simulink model "basic_test"  (R2013b) (continued)
== system basic_test == (continued)
      mask help:
      | Fold adders into DSPs: Causes adders to be absorbed into DSP blocks (supported in Virtex5)
      | Adder implementation: Cores using Fabric or DSP48 or behavioral HDL
      mask init: same as basic_test/pfb_fir_real0
      settings: AttributesFormatString=taps=4, add_latency=1
      mask Help: eval('xlWeb(''http://casper.berkeley.edu/wiki/Pfb_fir_real'')')
      mask Callback TotalTaps:
      | %num_taps = eval(get_param(gcb, 'TotalTaps'));
      | %old_vec = eval(get_param(gcb, 'mult_spec'));=-0l
      | 
      | %vec = 2.*ones(1,num_taps);
      | %old_size = length(old_vec);
      | 
      | %if(old_size <= num_taps),
      | %    new_size = old_size;
      | %else,
      | %    new_size = num_taps;
      | %end
      | 
      | %for n = 1:new_size, vec(n) = old_vec(n); end
      | %set_param(gcb, 'mult_spec', mat2str(vec));
      mask TypeOptions WindowType: bartlett | barthannwin | blackman | blackmanharris | bohamwin | chebwin | flattopwin | gausswin | hamming | hann | kaiser | nuttallwin | parzenwin | rectwin | tukeywin | triang | userwindow
      mask TypeOptions quantization: Truncate | Round  (unbiased: +/- Inf) | Round  (unbiased: Even Values)
      mask TypeOptions adder_imp: Behavioral | Fabric | DSP48
  pipeline : Subsystem  AncestorBlock=casper_library_delays/pipeline  mask(pipeline){latency=1}
      mask help:
      | An explicitly laid-out delay line to avoid mapping to SRL16s
      | for use in pipelining to help achieve timing closure.
      mask init:
      | pipeline_init(gcb,'latency',latency);
      settings: AttributesFormatString=Latency = %<latency>
      mask Display:
      | port_label('input', 1, 'd');
      | port_label('output', 1, 'q');
      | disp(['Z^-', num2str(latency)]);
  pipeline{1..7,9..12,14..21,25..29,93,256..263,275..276} (35 alike) : casper_library_delays/pipeline  latency=3
      settings: AttributesFormatString=Latency = %<latency>
  pipeline127 : Subsystem  AncestorBlock=casper_library_delays/pipeline  mask(pipeline){latency=2}
      mask help:
      | An explicitly laid-out delay line to avoid mapping to SRL16s
      | for use in pipelining to help achieve timing closure.
      mask init:
      | pipeline_init(gcb,'latency',latency);
      settings: AttributesFormatString=Latency = %<latency>
      mask Display:
      | port_label('input', 1, 'd');
      | port_label('output', 1, 'q');
      | disp(['Z^-', num2str(latency)]);
  pipeline128 : Subsystem  AncestorBlock=casper_library_delays/pipeline  mask(pipeline){latency=2}
      mask help:
      | An explicitly laid-out delay line to avoid mapping to SRL16s
      | for use in pipelining to help achieve timing closure.
      mask init:
      | pipeline_init(gcb,'latency',latency);
      settings: AttributesFormatString=Latency = %<latency>
      mask Display:
      | port_label('input', 1, 'd');
      | port_label('output', 1, 'q');
      | disp(['Z^-', num2str(latency)]);
  pipeline13 : Subsystem  AncestorBlock=casper_library_delays/pipeline  mask(pipeline){latency=1}
      mask help:
      | An explicitly laid-out delay line to avoid mapping to SRL16s
      | for use in pipelining to help achieve timing closure.
      mask init:
      | pipeline_init(gcb,'latency',latency);
      settings: AttributesFormatString=Latency = %<latency>
      mask Display:
      | port_label('input', 1, 'd');
      | port_label('output', 1, 'q');
      | disp(['Z^-', num2str(latency)]);
  pipeline22 : Subsystem  AncestorBlock=casper_library_delays/pipeline  mask(pipeline){latency=2}
      mask help:
      | An explicitly laid-out delay line to avoid mapping to SRL16s
      | for use in pipelining to help achieve timing closure.
      mask init:
      | pipeline_init(gcb,'latency',latency);
      settings: AttributesFormatString=Latency = %<latency>
      mask Display:
      | port_label('input', 1, 'd');
      | port_label('output', 1, 'q');
      | disp(['Z^-', num2str(latency)]);
  pipeline23 : Subsystem  AncestorBlock=casper_library_delays/pipeline  mask(pipeline){latency=1}
      mask help:
      | An explicitly laid-out delay line to avoid mapping to SRL16s
      | for use in pipelining to help achieve timing closure.
      mask init:
      | pipeline_init(gcb,'latency',latency);
      settings: AttributesFormatString=Latency = %<latency>
      mask Display:
      | port_label('input', 1, 'd');
      | port_label('output', 1, 'q');
      | disp(['Z^-', num2str(latency)]);
  pipeline24 : Subsystem  AncestorBlock=casper_library_delays/pipeline  mask(pipeline){latency=1}
      mask help:
      | An explicitly laid-out delay line to avoid mapping to SRL16s
      | for use in pipelining to help achieve timing closure.
      mask init:
      | pipeline_init(gcb,'latency',latency);
      settings: AttributesFormatString=Latency = %<latency>
      mask Display:
      | port_label('input', 1, 'd');
      | port_label('output', 1, 'q');
      | disp(['Z^-', num2str(latency)]);
  pipeline250 : Subsystem  AncestorBlock=casper_library_delays/pipeline  mask(pipeline){latency=1}
      mask help:
      | An explicitly laid-out delay line to avoid mapping to SRL16s
      | for use in pipelining to help achieve timing closure.
      mask init:
      | pipeline_init(gcb,'latency',latency);
      settings: AttributesFormatString=Latency = %<latency>
      mask Display:
      | port_label('input', 1, 'd');
      | port_label('output', 1, 'q');
      | disp(['Z^-', num2str(latency)]);
  pipeline251 : Subsystem  AncestorBlock=casper_library_delays/pipeline  mask(pipeline){latency=1}
      mask help:
      | An explicitly laid-out delay line to avoid mapping to SRL16s
      | for use in pipelining to help achieve timing closure.
      mask init:
      | pipeline_init(gcb,'latency',latency);
      settings: AttributesFormatString=Latency = %<latency>
      mask Display:
      | port_label('input', 1, 'd');
      | port_label('output', 1, 'q');
      | disp(['Z^-', num2str(latency)]);
  pipeline30 : Subsystem  AncestorBlock=casper_library_delays/pipeline  mask(pipeline){latency=1}
      mask help:
      | An explicitly laid-out delay line to avoid mapping to SRL16s
      | for use in pipelining to help achieve timing closure.
      mask init:
      | pipeline_init(gcb,'latency',latency);
      settings: AttributesFormatString=Latency = %<latency>
      mask Display:
      | port_label('input', 1, 'd');
      | port_label('output', 1, 'q');
      | disp(['Z^-', num2str(latency)]);
  pipeline56 : Subsystem  AncestorBlock=casper_library_delays/pipeline  mask(pipeline){latency=2}
      mask help:
      | An explicitly laid-out delay line to avoid mapping to SRL16s
      | for use in pipelining to help achieve timing closure.
      mask init:
      | pipeline_init(gcb,'latency',latency);
      settings: AttributesFormatString=Latency = %<latency>
      mask Display:
      | port_label('input', 1, 'd');
      | port_label('output', 1, 'q');
      | disp(['Z^-', num2str(latency)]);
  pipeline57 : Subsystem  AncestorBlock=casper_library_delays/pipeline  mask(pipeline){latency=2}
      mask help:
      | An explicitly laid-out delay line to avoid mapping to SRL16s
      | for use in pipelining to help achieve timing closure.
      mask init:
      | pipeline_init(gcb,'latency',latency);
      settings: AttributesFormatString=Latency = %<latency>
      mask Display:
      | port_label('input', 1, 'd');
      | port_label('output', 1, 'q');
      | disp(['Z^-', num2str(latency)]);
  pipeline58 : Subsystem  AncestorBlock=casper_library_delays/pipeline  mask(pipeline){latency=1}
      mask help:
      | An explicitly laid-out delay line to avoid mapping to SRL16s
      | for use in pipelining to help achieve timing closure.
      mask init:
      | pipeline_init(gcb,'latency',latency);
      settings: AttributesFormatString=Latency = %<latency>
      mask Display:
      | port_label('input', 1, 'd');
      | port_label('output', 1, 'q');
      | disp(['Z^-', num2str(latency)]);
  pipeline59 : Subsystem  AncestorBlock=casper_library_delays/pipeline  mask(pipeline){latency=1}
      mask help:
      | An explicitly laid-out delay line to avoid mapping to SRL16s
      | for use in pipelining to help achieve timing closure.
      mask init:
      | pipeline_init(gcb,'latency',latency);
      settings: AttributesFormatString=Latency = %<latency>
      mask Display:
      | port_label('input', 1, 'd');
      | port_label('output', 1, 'q');
      | disp(['Z^-', num2str(latency)]);
  pipeline60 : Subsystem  AncestorBlock=casper_library_delays/pipeline  mask(pipeline){latency=1}
      mask help:
      | An explicitly laid-out delay line to avoid mapping to SRL16s
      | for use in pipelining to help achieve timing closure.
      mask init:
      | pipeline_init(gcb,'latency',latency);
      settings: AttributesFormatString=Latency = %<latency>
      mask Display:
      | port_label('input', 1, 'd');
      | port_label('output', 1, 'q');
      | disp(['Z^-', num2str(latency)]);
  pipeline61 : Subsystem  AncestorBlock=casper_library_delays/pipeline  mask(pipeline){latency=2}
      mask help:
      | An explicitly laid-out delay line to avoid mapping to SRL16s
      | for use in pipelining to help achieve timing closure.
      mask init:
      | pipeline_init(gcb,'latency',latency);
      settings: AttributesFormatString=Latency = %<latency>
      mask Display:
      | port_label('input', 1, 'd');
      | port_label('output', 1, 'q');
      | disp(['Z^-', num2str(latency)]);
  pipeline62 : Subsystem  AncestorBlock=casper_library_delays/pipeline  mask(pipeline){latency=2}
      mask help:
      | An explicitly laid-out delay line to avoid mapping to SRL16s
      | for use in pipelining to help achieve timing closure.
      mask init:
      | pipeline_init(gcb,'latency',latency);
      settings: AttributesFormatString=Latency = %<latency>
      mask Display:
      | port_label('input', 1, 'd');
      | port_label('output', 1, 'q');
      | disp(['Z^-', num2str(latency)]);
  pipeline63 : Subsystem  AncestorBlock=casper_library_delays/pipeline  mask(pipeline){latency=1}
      mask help:
      | An explicitly laid-out delay line to avoid mapping to SRL16s
      | for use in pipelining to help achieve timing closure.
      mask init:
      | pipeline_init(gcb,'latency',latency);
      settings: AttributesFormatString=Latency = %<latency>
      mask Display:
      | port_label('input', 1, 'd');
      | port_label('output', 1, 'q');
      | disp(['Z^-', num2str(latency)]);
  pipeline64 : Subsystem  AncestorBlock=casper_library_delays/pipeline  mask(pipeline){latency=1}
      mask help:
      | An explicitly laid-out delay line to avoid mapping to SRL16s
      | for use in pipelining to help achieve timing closure.
      mask init:
      | pipeline_init(gcb,'latency',latency);
      settings: AttributesFormatString=Latency = %<latency>
      mask Display:
      | port_label('input', 1, 'd');
      | port_label('output', 1, 'q');
      | disp(['Z^-', num2str(latency)]);
  pipeline65 : Subsystem  AncestorBlock=casper_library_delays/pipeline  mask(pipeline){latency=2}
      mask help:
      | An explicitly laid-out delay line to avoid mapping to SRL16s
      | for use in pipelining to help achieve timing closure.
      mask init:
      | pipeline_init(gcb,'latency',latency);
      settings: AttributesFormatString=Latency = %<latency>
      mask Display:
      | port_label('input', 1, 'd');
      | port_label('output', 1, 'q');
      | disp(['Z^-', num2str(latency)]);
  pipeline66 : Subsystem  AncestorBlock=casper_library_delays/pipeline  mask(pipeline){latency=2}
      mask help:
      | An explicitly laid-out delay line to avoid mapping to SRL16s
      | for use in pipelining to help achieve timing closure.
      mask init:
      | pipeline_init(gcb,'latency',latency);
      settings: AttributesFormatString=Latency = %<latency>
      mask Display:
      | port_label('input', 1, 'd');
      | port_label('output', 1, 'q');
      | disp(['Z^-', num2str(latency)]);
  pipeline67 : Subsystem  AncestorBlock=casper_library_delays/pipeline  mask(pipeline){latency=1}
      mask help:
      | An explicitly laid-out delay line to avoid mapping to SRL16s
      | for use in pipelining to help achieve timing closure.
      mask init:
      | pipeline_init(gcb,'latency',latency);
      settings: AttributesFormatString=Latency = %<latency>
      mask Display:
      | port_label('input', 1, 'd');
      | port_label('output', 1, 'q');
      | disp(['Z^-', num2str(latency)]);
  pipeline68 : Subsystem  AncestorBlock=casper_library_delays/pipeline  mask(pipeline){latency=1}
      mask help:
      | An explicitly laid-out delay line to avoid mapping to SRL16s
      | for use in pipelining to help achieve timing closure.
      mask init:
      | pipeline_init(gcb,'latency',latency);
      settings: AttributesFormatString=Latency = %<latency>
      mask Display:
      | port_label('input', 1, 'd');
      | port_label('output', 1, 'q');
      | disp(['Z^-', num2str(latency)]);
  pipeline69 : Subsystem  AncestorBlock=casper_library_delays/pipeline  mask(pipeline){latency=2}
      mask help:
      | An explicitly laid-out delay line to avoid mapping to SRL16s
      | for use in pipelining to help achieve timing closure.
      mask init:
      | pipeline_init(gcb,'latency',latency);
      settings: AttributesFormatString=Latency = %<latency>
      mask Display:
      | port_label('input', 1, 'd');
      | port_label('output', 1, 'q');
      | disp(['Z^-', num2str(latency)]);
  pipeline70 : Subsystem  AncestorBlock=casper_library_delays/pipeline  mask(pipeline){latency=2}
      mask help:
      | An explicitly laid-out delay line to avoid mapping to SRL16s
      | for use in pipelining to help achieve timing closure.
      mask init:
      | pipeline_init(gcb,'latency',latency);
      settings: AttributesFormatString=Latency = %<latency>
      mask Display:
      | port_label('input', 1, 'd');
      | port_label('output', 1, 'q');
      | disp(['Z^-', num2str(latency)]);
  pipeline71 : Subsystem  AncestorBlock=casper_library_delays/pipeline  mask(pipeline){latency=1}
      mask help:
      | An explicitly laid-out delay line to avoid mapping to SRL16s
      | for use in pipelining to help achieve timing closure.
      mask init:
      | pipeline_init(gcb,'latency',latency);
      settings: AttributesFormatString=Latency = %<latency>
      mask Display:
      | port_label('input', 1, 'd');
      | port_label('output', 1, 'q');
      | disp(['Z^-', num2str(latency)]);
  pipeline72 : Subsystem  AncestorBlock=casper_library_delays/pipeline  mask(pipeline){latency=5}
      mask help:
      | An explicitly laid-out delay line to avoid mapping to SRL16s
      | for use in pipelining to help achieve timing closure.
      mask init:
      | pipeline_init(gcb,'latency',latency);
      settings: AttributesFormatString=Latency = %<latency>
      mask Display:
      | port_label('input', 1, 'd');
      | port_label('output', 1, 'q');
      | disp(['Z^-', num2str(latency)]);
  pipeline73 : Subsystem  AncestorBlock=casper_library_delays/pipeline  mask(pipeline){latency=1}
      mask help:
      | An explicitly laid-out delay line to avoid mapping to SRL16s
      | for use in pipelining to help achieve timing closure.
      mask init:
      | pipeline_init(gcb,'latency',latency);
      settings: AttributesFormatString=Latency = %<latency>
      mask Display:
      | port_label('input', 1, 'd');
      | port_label('output', 1, 'q');
      | disp(['Z^-', num2str(latency)]);
  pipeline74 : Subsystem  AncestorBlock=casper_library_delays/pipeline  mask(pipeline){latency=2}
      mask help:
      | An explicitly laid-out delay line to avoid mapping to SRL16s
      | for use in pipelining to help achieve timing closure.
      mask init:
      | pipeline_init(gcb,'latency',latency);
      settings: AttributesFormatString=Latency = %<latency>
      mask Display:
      | port_label('input', 1, 'd');
      | port_label('output', 1, 'q');
      | disp(['Z^-', num2str(latency)]);
  pipeline75 : Subsystem  AncestorBlock=casper_library_delays/pipeline  mask(pipeline){latency=2}
      mask help:
      | An explicitly laid-out delay line to avoid mapping to SRL16s
      | for use in pipelining to help achieve timing closure.
      mask init:
      | pipeline_init(gcb,'latency',latency);
      settings: AttributesFormatString=Latency = %<latency>
      mask Display:
      | port_label('input', 1, 'd');
      | port_label('output', 1, 'q');
      | disp(['Z^-', num2str(latency)]);
  pipeline76 : Subsystem  AncestorBlock=casper_library_delays/pipeline  mask(pipeline){latency=1}
      mask help:
      | An explicitly laid-out delay line to avoid mapping to SRL16s
      | for use in pipelining to help achieve timing closure.
      mask init:
      | pipeline_init(gcb,'latency',latency);
      settings: AttributesFormatString=Latency = %<latency>
      mask Display:
      | port_label('input', 1, 'd');
      | port_label('output', 1, 'q');
      | disp(['Z^-', num2str(latency)]);
  pipeline77 : Subsystem  AncestorBlock=casper_library_delays/pipeline  mask(pipeline){latency=1}
      mask help:
      | An explicitly laid-out delay line to avoid mapping to SRL16s
      | for use in pipelining to help achieve timing closure.
      mask init:
      | pipeline_init(gcb,'latency',latency);
      settings: AttributesFormatString=Latency = %<latency>
      mask Display:
      | port_label('input', 1, 'd');
      | port_label('output', 1, 'q');
      | disp(['Z^-', num2str(latency)]);
  pipeline78 : Subsystem  AncestorBlock=casper_library_delays/pipeline  mask(pipeline){latency=1}
      mask help:
      | An explicitly laid-out delay line to avoid mapping to SRL16s
      | for use in pipelining to help achieve timing closure.
      mask init:
      | pipeline_init(gcb,'latency',latency);
      settings: AttributesFormatString=Latency = %<latency>
      mask Display:
      | port_label('input', 1, 'd');
      | port_label('output', 1, 'q');
      | disp(['Z^-', num2str(latency)]);
  pipeline79 : Subsystem  AncestorBlock=casper_library_delays/pipeline  mask(pipeline){latency=2}
      mask help:
      | An explicitly laid-out delay line to avoid mapping to SRL16s
      | for use in pipelining to help achieve timing closure.
      mask init:
      | pipeline_init(gcb,'latency',latency);
      settings: AttributesFormatString=Latency = %<latency>
      mask Display:
      | port_label('input', 1, 'd');
      | port_label('output', 1, 'q');
      | disp(['Z^-', num2str(latency)]);
  pipeline8 : Subsystem  AncestorBlock=casper_library_delays/pipeline  mask(pipeline){latency=1}
      mask help:
      | An explicitly laid-out delay line to avoid mapping to SRL16s
      | for use in pipelining to help achieve timing closure.
      mask init:
      | pipeline_init(gcb,'latency',latency);
      settings: AttributesFormatString=Latency = %<latency>
      mask Display:
      | port_label('input', 1, 'd');
      | port_label('output', 1, 'q');
      | disp(['Z^-', num2str(latency)]);
  pipeline80 : Subsystem  AncestorBlock=casper_library_delays/pipeline  mask(pipeline){latency=2}
      mask help:
      | An explicitly laid-out delay line to avoid mapping to SRL16s
      | for use in pipelining to help achieve timing closure.
      mask init:
      | pipeline_init(gcb,'latency',latency);
      settings: AttributesFormatString=Latency = %<latency>
      mask Display:
      | port_label('input', 1, 'd');
      | port_label('output', 1, 'q');
      | disp(['Z^-', num2str(latency)]);
  pipeline81 : Subsystem  AncestorBlock=casper_library_delays/pipeline  mask(pipeline){latency=1}
      mask help:
      | An explicitly laid-out delay line to avoid mapping to SRL16s
      | for use in pipelining to help achieve timing closure.
      mask init:
      | pipeline_init(gcb,'latency',latency);
      settings: AttributesFormatString=Latency = %<latency>
      mask Display:
      | port_label('input', 1, 'd');
      | port_label('output', 1, 'q');
      | disp(['Z^-', num2str(latency)]);
  pipeline82 : Subsystem  AncestorBlock=casper_library_delays/pipeline  mask(pipeline){latency=1}
      mask help:
      | An explicitly laid-out delay line to avoid mapping to SRL16s
      | for use in pipelining to help achieve timing closure.
      mask init:
      | pipeline_init(gcb,'latency',latency);
      settings: AttributesFormatString=Latency = %<latency>
      mask Display:
      | port_label('input', 1, 'd');
      | port_label('output', 1, 'q');
      | disp(['Z^-', num2str(latency)]);
  pipeline83 : Subsystem  AncestorBlock=casper_library_delays/pipeline  mask(pipeline){latency=2}
      mask help:
      | An explicitly laid-out delay line to avoid mapping to SRL16s
      | for use in pipelining to help achieve timing closure.
      mask init:
      | pipeline_init(gcb,'latency',latency);
      settings: AttributesFormatString=Latency = %<latency>
      mask Display:
      | port_label('input', 1, 'd');
      | port_label('output', 1, 'q');
      | disp(['Z^-', num2str(latency)]);
  pipeline84 : Subsystem  AncestorBlock=casper_library_delays/pipeline  mask(pipeline){latency=2}
      mask help:
      | An explicitly laid-out delay line to avoid mapping to SRL16s
      | for use in pipelining to help achieve timing closure.
      mask init:
      | pipeline_init(gcb,'latency',latency);
      settings: AttributesFormatString=Latency = %<latency>
      mask Display:
      | port_label('input', 1, 'd');
      | port_label('output', 1, 'q');
      | disp(['Z^-', num2str(latency)]);
  pipeline85 : Subsystem  AncestorBlock=casper_library_delays/pipeline  mask(pipeline){latency=1}
      mask help:
      | An explicitly laid-out delay line to avoid mapping to SRL16s
      | for use in pipelining to help achieve timing closure.
      mask init:
      | pipeline_init(gcb,'latency',latency);
      settings: AttributesFormatString=Latency = %<latency>
      mask Display:
      | port_label('input', 1, 'd');
      | port_label('output', 1, 'q');
      | disp(['Z^-', num2str(latency)]);
  pipeline86 : Subsystem  AncestorBlock=casper_library_delays/pipeline  mask(pipeline){latency=1}
      mask help:
      | An explicitly laid-out delay line to avoid mapping to SRL16s
      | for use in pipelining to help achieve timing closure.
      mask init:
      | pipeline_init(gcb,'latency',latency);
      settings: AttributesFormatString=Latency = %<latency>
      mask Display:
      | port_label('input', 1, 'd');
      | port_label('output', 1, 'q');
      | disp(['Z^-', num2str(latency)]);
  pipeline87 : Subsystem  AncestorBlock=casper_library_delays/pipeline  mask(pipeline){latency=2}
      mask help:
      | An explicitly laid-out delay line to avoid mapping to SRL16s
      | for use in pipelining to help achieve timing closure.
      mask init:
      | pipeline_init(gcb,'latency',latency);
      settings: AttributesFormatString=Latency = %<latency>
      mask Display:
      | port_label('input', 1, 'd');
      | port_label('output', 1, 'q');
      | disp(['Z^-', num2str(latency)]);
  pipeline88 : Subsystem  AncestorBlock=casper_library_delays/pipeline  mask(pipeline){latency=2}
      mask help:
      | An explicitly laid-out delay line to avoid mapping to SRL16s
      | for use in pipelining to help achieve timing closure.
      mask init:
      | pipeline_init(gcb,'latency',latency);
      settings: AttributesFormatString=Latency = %<latency>
      mask Display:
      | port_label('input', 1, 'd');
      | port_label('output', 1, 'q');
      | disp(['Z^-', num2str(latency)]);
  pipeline89 : Subsystem  AncestorBlock=casper_library_delays/pipeline  mask(pipeline){latency=1}
      mask help:
      | An explicitly laid-out delay line to avoid mapping to SRL16s
      | for use in pipelining to help achieve timing closure.
      mask init:
      | pipeline_init(gcb,'latency',latency);
      settings: AttributesFormatString=Latency = %<latency>
      mask Display:
      | port_label('input', 1, 'd');
      | port_label('output', 1, 'q');
      | disp(['Z^-', num2str(latency)]);
  pipeline90 : Subsystem  AncestorBlock=casper_library_delays/pipeline  mask(pipeline){latency=5}
      mask help:
      | An explicitly laid-out delay line to avoid mapping to SRL16s
      | for use in pipelining to help achieve timing closure.
      mask init:
      | pipeline_init(gcb,'latency',latency);
      settings: AttributesFormatString=Latency = %<latency>
      mask Display:
      | port_label('input', 1, 'd');
      | port_label('output', 1, 'q');
      | disp(['Z^-', num2str(latency)]);
  pipeline91 : Subsystem  AncestorBlock=casper_library_delays/pipeline  mask(pipeline){latency=1}
      mask help:
      | An explicitly laid-out delay line to avoid mapping to SRL16s
      | for use in pipelining to help achieve timing closure.
      mask init:
      | pipeline_init(gcb,'latency',latency);
      settings: AttributesFormatString=Latency = %<latency>
      mask Display:
      | port_label('input', 1, 'd');
      | port_label('output', 1, 'q');
      | disp(['Z^-', num2str(latency)]);
  pipeline92 : Subsystem  AncestorBlock=casper_library_delays/pipeline  mask(pipeline){latency=2}
      mask help:
      | An explicitly laid-out delay line to avoid mapping to SRL16s
      | for use in pipelining to help achieve timing closure.
      mask init:
      | pipeline_init(gcb,'latency',latency);
      settings: AttributesFormatString=Latency = %<latency>
      mask Display:
      | port_label('input', 1, 'd');
      | port_label('output', 1, 'q');
      | disp(['Z^-', num2str(latency)]);
  power{1..7,16} (8 alike) : casper_library_misc/power  BitWidth=18  add_latency=2  mult_latency=3
  power_convert0 : Subsystem  mask{shift_val=1, conv_width=20, conv_point=15}
      mask prompts: shift_val "shift"; conv_width "convert width"; conv_point "convert point"
  power_convert1 : Subsystem  mask{shift_val=1, conv_width=20, conv_point=15}
      mask prompts: shift_val "shift"; conv_width "convert width"; conv_point "convert point"
  r11 : Subsystem  Tag=xps:bram  AncestorBlock=xps_library/Shared_BRAM  mask{arith_type=Unsigned, addr_width=10, data_width=32, reg_prim_output=off, reg_core_output=off, optimization=Minimum_Area, data_bin_pt=0, init_vals=[0:2^10-1], sample_rate=1}
      mask prompts: arith_type "Output Data Type"; addr_width "Address width"; data_width "Data Width"; reg_prim_output "Register Primitive Output"; reg_core_output "Register Core Output"; data_bin_pt "Data Binary Point"; init_vals "Initial values (simulation only)"; sample_rate "Sample rate"
      mask init:
      | shared_bram_mask;
      mask TypeOptions arith_type: Unsigned | Signed  (2's comp)
      mask TypeOptions data_width: 8 | 16 | 32 | 64 | 128
      mask TypeOptions optimization: Minimum_Area | Low_Power
  r12 : Subsystem  Tag=xps:bram  AncestorBlock=xps_library/Shared_BRAM  mask{arith_type=Unsigned, addr_width=10, data_width=32, reg_prim_output=off, reg_core_output=off, optimization=Minimum_Area, data_bin_pt=0, init_vals=[0:2^10-1], sample_rate=1}
      mask prompts: arith_type "Output Data Type"; addr_width "Address width"; data_width "Data Width"; reg_prim_output "Register Primitive Output"; reg_core_output "Register Core Output"; data_bin_pt "Data Binary Point"; init_vals "Initial values (simulation only)"; sample_rate "Sample rate"
      mask init:
      | shared_bram_mask;
      mask TypeOptions arith_type: Unsigned | Signed  (2's comp)
      mask TypeOptions data_width: 8 | 16 | 32 | 64 | 128
      mask TypeOptions optimization: Minimum_Area | Low_Power
  r22 : Subsystem  Tag=xps:bram  AncestorBlock=xps_library/Shared_BRAM  mask{arith_type=Unsigned, addr_width=10, data_width=32, reg_prim_output=off, reg_core_output=off, optimization=Minimum_Area, data_bin_pt=0, init_vals=[0:2^10-1], sample_rate=1}
      mask prompts: arith_type "Output Data Type"; addr_width "Address width"; data_width "Data Width"; reg_prim_output "Register Primitive Output"; reg_core_output "Register Core Output"; data_bin_pt "Data Binary Point"; init_vals "Initial values (simulation only)"; sample_rate "Sample rate"
      mask init:
      | shared_bram_mask;
      mask TypeOptions arith_type: Unsigned | Signed  (2's comp)
      mask TypeOptions data_width: 8 | 16 | 32 | 64 | 128
      mask TypeOptions optimization: Minimum_Area | Low_Power
  simple_bram_vacc_0_0 : Subsystem  AncestorBlock=casper_library_accumulators/simple_bram_vacc  mask(simple_bram_vacc){vec_len=512, arith_type=Unsigned, n_bits=64, bin_pt=35}
      mask prompts: vec_len "Vector length:"; arith_type "Output type:"; n_bits "Bit width (output):"; bin_pt "Binary point (output):"
      mask help: This simple vector accumulator is based on a shift register. It outputs its previous accumulated vector after a new_acc pulse is received.
      mask init:
      | simple_bram_vacc_init(gcb, ...
      |     'vec_len', vec_len, ...
      |     'arith_type', arith_type, ...
      |     'n_bits', n_bits, ...
      |     'bin_pt', bin_pt);
      mask TypeOptions arith_type: Signed | Unsigned
  simple_bram_vacc_0_1 : Subsystem  AncestorBlock=casper_library_accumulators/simple_bram_vacc  mask(simple_bram_vacc){vec_len=512, arith_type=Unsigned, n_bits=64, bin_pt=35}
      mask prompts: vec_len "Vector length:"; arith_type "Output type:"; n_bits "Bit width (output):"; bin_pt "Binary point (output):"
      mask help: This simple vector accumulator is based on a shift register. It outputs its previous accumulated vector after a new_acc pulse is received.
      mask init:
      | simple_bram_vacc_init(gcb, ...
      |     'vec_len', vec_len, ...
      |     'arith_type', arith_type, ...
      |     'n_bits', n_bits, ...
      |     'bin_pt', bin_pt);
      mask TypeOptions arith_type: Signed | Unsigned
  simple_bram_vacc_0_2 : Subsystem  AncestorBlock=casper_library_accumulators/simple_bram_vacc  mask(simple_bram_vacc){vec_len=512, arith_type=Unsigned, n_bits=64, bin_pt=35}
      mask prompts: vec_len "Vector length:"; arith_type "Output type:"; n_bits "Bit width (output):"; bin_pt "Binary point (output):"
      mask help: This simple vector accumulator is based on a shift register. It outputs its previous accumulated vector after a new_acc pulse is received.
      mask init:
      | simple_bram_vacc_init(gcb, ...
      |     'vec_len', vec_len, ...
      |     'arith_type', arith_type, ...
      |     'n_bits', n_bits, ...
      |     'bin_pt', bin_pt);
      mask TypeOptions arith_type: Signed | Unsigned
  simple_bram_vacc_0_3 : Subsystem  AncestorBlock=casper_library_accumulators/simple_bram_vacc  mask(simple_bram_vacc){vec_len=512, arith_type=Unsigned, n_bits=64, bin_pt=35}
      mask prompts: vec_len "Vector length:"; arith_type "Output type:"; n_bits "Bit width (output):"; bin_pt "Binary point (output):"
      mask help: This simple vector accumulator is based on a shift register. It outputs its previous accumulated vector after a new_acc pulse is received.
      mask init:
      | simple_bram_vacc_init(gcb, ...
      |     'vec_len', vec_len, ...
      |     'arith_type', arith_type, ...
      |     'n_bits', n_bits, ...
      |     'bin_pt', bin_pt);
      mask TypeOptions arith_type: Signed | Unsigned
  simple_bram_vacc_0_4 : Subsystem  AncestorBlock=casper_library_accumulators/simple_bram_vacc  mask(simple_bram_vacc){vec_len=512, arith_type=Unsigned, n_bits=64, bin_pt=35}
      mask prompts: vec_len "Vector length:"; arith_type "Output type:"; n_bits "Bit width (output):"; bin_pt "Binary point (output):"
      mask help: This simple vector accumulator is based on a shift register. It outputs its previous accumulated vector after a new_acc pulse is received.
      mask init:
      | simple_bram_vacc_init(gcb, ...
      |     'vec_len', vec_len, ...
      |     'arith_type', arith_type, ...
      |     'n_bits', n_bits, ...
      |     'bin_pt', bin_pt);
      mask TypeOptions arith_type: Signed | Unsigned
  simple_bram_vacc_0_5 : Subsystem  AncestorBlock=casper_library_accumulators/simple_bram_vacc  mask(simple_bram_vacc){vec_len=512, arith_type=Unsigned, n_bits=64, bin_pt=35}
      mask prompts: vec_len "Vector length:"; arith_type "Output type:"; n_bits "Bit width (output):"; bin_pt "Binary point (output):"
      mask help: This simple vector accumulator is based on a shift register. It outputs its previous accumulated vector after a new_acc pulse is received.
      mask init:
      | simple_bram_vacc_init(gcb, ...
      |     'vec_len', vec_len, ...
      |     'arith_type', arith_type, ...
      |     'n_bits', n_bits, ...
      |     'bin_pt', bin_pt);
      mask TypeOptions arith_type: Signed | Unsigned
  simple_bram_vacc_0_6 : Subsystem  AncestorBlock=casper_library_accumulators/simple_bram_vacc  mask(simple_bram_vacc){vec_len=512, arith_type=Unsigned, n_bits=64, bin_pt=35}
      mask prompts: vec_len "Vector length:"; arith_type "Output type:"; n_bits "Bit width (output):"; bin_pt "Binary point (output):"
      mask help: This simple vector accumulator is based on a shift register. It outputs its previous accumulated vector after a new_acc pulse is received.
      mask init:
      | simple_bram_vacc_init(gcb, ...
      |     'vec_len', vec_len, ...
      |     'arith_type', arith_type, ...
      |     'n_bits', n_bits, ...
      |     'bin_pt', bin_pt);
      mask TypeOptions arith_type: Signed | Unsigned
  simple_bram_vacc_0_7 : Subsystem  AncestorBlock=casper_library_accumulators/simple_bram_vacc  mask(simple_bram_vacc){vec_len=512, arith_type=Unsigned, n_bits=64, bin_pt=35}
      mask prompts: vec_len "Vector length:"; arith_type "Output type:"; n_bits "Bit width (output):"; bin_pt "Binary point (output):"
      mask help: This simple vector accumulator is based on a shift register. It outputs its previous accumulated vector after a new_acc pulse is received.
      mask init:
      | simple_bram_vacc_init(gcb, ...
      |     'vec_len', vec_len, ...
      |     'arith_type', arith_type, ...
      |     'n_bits', n_bits, ...
      |     'bin_pt', bin_pt);
      mask TypeOptions arith_type: Signed | Unsigned
  sync_gen : Subsystem  AncestorBlock=casper_library_misc/sync_gen  mask(sync_gen){sim_acc_len=1, fft_size=4096, fft_simult_inputs=8, pfb_fir_taps=4, reorder_vec=[2 2 9], scale=1}
      mask prompts: sim_acc_len "Simulation Accumulation Length"; fft_size "FFT Size"; fft_simult_inputs "Simultaneous Inputs (FFT)"; pfb_fir_taps "Taps in pfb_fir (use 1 if no pfb_fir)"; reorder_vec "Reorder Orders (e.g. [2 2 2])"
      mask help:
      | This block computes the sync period based on system parameters as
      | described in Casper memo.  Detailed descritions of the block's
      | variables can be found in the 'Block Documentation' section of
      | the CASPER wiki.
      mask init:
      | sync_gen_init(gcb, ...
      |     'sim_acc_len', sim_acc_len, ...
      |     'fft_size', fft_size, ...
      |     'fft_simult_inputs', fft_simult_inputs,...
      |     'pfb_fir_taps', pfb_fir_taps, ...
      |     'reorder_vec', reorder_vec, ...
      |     'scale', scale);
      settings: AttributesFormatString=sim_sync_period=36864
      mask Help:
      | SyncPeriod=LCM(reorder_vec)*(acc_num)*(pfb_fir_len)*(fft_size/fft_simult_inputs)
      | 
      | See CASPER sync pulse memo for more details.
  wires:
  fft_wideband_real0/6(of) -> Terminator1/1
  bus_create/1(bus_out) -> pipeline276/1
  acc_len/1(in_reg) -> pipeline250/1(d)
  cnt_rst/1(in_reg) -> pipeline251/1(d)
  pipeline250/1(q) -> [acc_len]
  pipeline251/1(q) -> Slice1/1
  Band-Limited White Noise/1 -> asiaa_adc5g0/1(sim_a)
  Constant13/1 -> asiaa_adc5g0/3(sim_sync)
  sync_gen/1(sync_out) -> [sync_out]
  pipeline262/1 -> pfb_fir_real0/8(pol1_in7)
  pipeline257/1 -> pfb_fir_real0/3(pol1_in2)
  pipeline261/1 -> pfb_fir_real0/7(pol1_in6)
  pipeline260/1 -> pfb_fir_real0/6(pol1_in5)
  pipeline259/1 -> pfb_fir_real0/5(pol1_in4)
  pipeline258/1 -> pfb_fir_real0/4(pol1_in3)
  pipeline263/1 -> pfb_fir_real0/9(pol1_in8)
  pipeline256/1 -> pfb_fir_real0/2(pol1_in1)
  pipeline16/1 -> pfb_fir_real0/1(sync)
  pfb_fir_real0/1(sync_out) -> Delay15/1
  Delay15/1 -> fft_wideband_real0/1(sync)
  fft_wideband_real0/1(sync_out) -> pipeline275/1
  fft_wideband_real0/2(out00) -> pipeline1/1
  fft_wideband_real0/3(out01) -> pipeline2/1
  fft_wideband_real0/4(out02) -> pipeline3/1
  fft_wideband_real0/5(out03) -> pipeline9/1
  pipeline276/1 -> adcsnap0/1(din)
  Constant4/1 -> adcsnap0/3(trig), adcsnap0/2(we)
  Slice1/1 -> [cnt_rst]
  Band-Limited White Noise2/1 -> asiaa_adc5g0/2(sim_c)
  asiaa_adc5g0/17(sync_out) -> Terminator/1
  asiaa_adc5g0/1(a0) -> conv_bus0/1(i0)
  asiaa_adc5g0/2(a1) -> conv_bus0/2(i1)
  asiaa_adc5g0/3(a2) -> conv_bus0/3(i2)
  asiaa_adc5g0/4(a3) -> conv_bus0/4(i3)
  asiaa_adc5g0/5(a4) -> conv_bus0/5(i4)
  asiaa_adc5g0/6(a5) -> conv_bus0/6(i5)
  asiaa_adc5g0/7(a6) -> conv_bus0/7(i6)
  asiaa_adc5g0/8(a7) -> conv_bus0/8(i7)
  conv_bus0/7(q6) -> bus_create/7(in7), pipeline262/1
  conv_bus0/5(q4) -> bus_create/5(in5), pipeline260/1
  conv_bus0/4(q3) -> bus_create/4(in4), pipeline259/1
  conv_bus0/3(q2) -> bus_create/3(in3), pipeline258/1
  conv_bus0/2(q1) -> bus_create/2(in2), pipeline257/1
  conv_bus0/1(q0) -> bus_create/1(in1), pipeline256/1
  bus_create1/1(bus_out) -> pipeline4/1
  pipeline4/1 -> adcsnap1/1(din)
  Constant8/1 -> adcsnap1/3(trig), adcsnap1/2(we)
  conv_bus0/8(q7) -> bus_create/8(in8), pipeline263/1
  [sync_out] -> pipeline16/1
  [cnt_rst] -> acc_cntrl0/3(rst)
  [acc_len] -> acc_cntrl0/1(acc_len)
  dout_0a_0/1(data_out) -> Terminator9/1
  Reinterpret31/1 -> dout_0a_0/2(data_in)
  simple_bram_vacc_0_0/1(dout) -> pipeline74/1(d)
  simple_bram_vacc_0_0/2(valid) -> pipeline56/1(d)
  pipeline73/1(q) -> simple_bram_vacc_0_0/2(din)
  pipeline59/1(q) -> pipeline57/1(d)
  pipeline74/1(q) -> Reinterpret31/1
  pipeline56/1(q) -> Counter1/2, dout_0a_0/3(we)
  pipeline57/1(q) -> Counter1/1
  pipeline58/1(q) -> simple_bram_vacc_0_0/1(new_acc)
  dout_0a_1/1(data_out) -> Terminator6/1
  Reinterpret24/1 -> dout_0a_1/2(data_in)
  simple_bram_vacc_0_1/1(dout) -> pipeline61/1(d)
  simple_bram_vacc_0_1/2(valid) -> pipeline62/1(d)
  pipeline60/1(q) -> simple_bram_vacc_0_1/2(din)
  pipeline61/1(q) -> Reinterpret24/1
  pipeline62/1(q) -> dout_0a_1/3(we)
  pipeline63/1(q) -> simple_bram_vacc_0_1/1(new_acc)
  dout_0a_2/1(data_out) -> Terminator7/1
  Reinterpret25/1 -> dout_0a_2/2(data_in)
  simple_bram_vacc_0_2/1(dout) -> pipeline65/1(d)
  simple_bram_vacc_0_2/2(valid) -> pipeline66/1(d)
  pipeline64/1(q) -> simple_bram_vacc_0_2/2(din)
  pipeline65/1(q) -> Reinterpret25/1
  pipeline66/1(q) -> dout_0a_2/3(we)
  pipeline67/1(q) -> simple_bram_vacc_0_2/1(new_acc)
  dout_0a_3/1(data_out) -> Terminator8/1
  Reinterpret26/1 -> dout_0a_3/2(data_in)
  simple_bram_vacc_0_3/1(dout) -> pipeline69/1(d)
  simple_bram_vacc_0_3/2(valid) -> pipeline70/1(d)
  pipeline68/1(q) -> simple_bram_vacc_0_3/2(din)
  pipeline69/1(q) -> Reinterpret26/1
  pipeline70/1(q) -> dout_0a_3/3(we)
  pipeline71/1(q) -> simple_bram_vacc_0_3/1(new_acc)
  Delay32/1 -> pipeline73/1(d)
  Delay34/1 -> pipeline60/1(d)
  Delay35/1 -> pipeline64/1(d)
  Delay36/1 -> pipeline68/1(d)
  Counter1/1 -> dout_0a_1/1(addr), dout_0a_2/1(addr), dout_0a_3/1(addr), dout_0a_0/1(addr)
  acc_cntrl0/1(new_acc) -> pipeline63/1(d), pipeline71/1(d), pipeline67/1(d), pipeline58/1(d), pipeline59/1(d)
  pipeline275/1 -> pipeline72/1(d), [fft0_sync]
  pipeline1/1 -> [fft0_0], power16/1
  pipeline2/1 -> [fft0_1], power1/1
  pipeline3/1 -> [fft0_2], power2/1
  pipeline9/1 -> [fft0_3], power3/1
  Constant10/1 -> fft_wideband_real1/2(shift)
  fft_wideband_real1/6(of) -> Terminator5/1
  pipeline18/1 -> pfb_fir_real1/8(pol1_in7)
  pipeline11/1 -> pfb_fir_real1/3(pol1_in2)
  pipeline17/1 -> pfb_fir_real1/7(pol1_in6)
  pipeline15/1 -> pfb_fir_real1/6(pol1_in5)
  pipeline14/1 -> pfb_fir_real1/5(pol1_in4)
  pipeline12/1 -> pfb_fir_real1/4(pol1_in3)
  pipeline19/1 -> pfb_fir_real1/9(pol1_in8)
  pipeline10/1 -> pfb_fir_real1/2(pol1_in1)
  pipeline6/1 -> pfb_fir_real1/1(sync)
  pfb_fir_real1/1(sync_out) -> Delay3/1
  Delay3/1 -> fft_wideband_real1/1(sync)
  fft_wideband_real1/1(sync_out) -> pipeline20/1
  fft_wideband_real1/2(out00) -> pipeline5/1
  fft_wideband_real1/3(out01) -> pipeline7/1
  fft_wideband_real1/4(out02) -> pipeline21/1
  fft_wideband_real1/5(out03) -> pipeline93/1
  conv_bus1/7(q6) -> pipeline18/1, bus_create1/7(in7)
  conv_bus1/6(q5) -> bus_create1/6(in6), pipeline17/1
  conv_bus1/5(q4) -> bus_create1/5(in5), pipeline15/1
  conv_bus1/4(q3) -> bus_create1/4(in4), pipeline14/1
  conv_bus1/3(q2) -> bus_create1/3(in3), pipeline12/1
  conv_bus1/2(q1) -> bus_create1/2(in2), pipeline11/1
  conv_bus1/1(q0) -> bus_create1/1(in1), pipeline10/1
  conv_bus1/8(q7) -> bus_create1/8(in8), pipeline19/1
  [sync_out] -> pipeline6/1
  [cnt_rst] -> acc_cntrl1/3(rst)
  [acc_len] -> acc_cntrl1/1(acc_len)
  dout_0c_0/1(data_out) -> Terminator13/1
  Reinterpret4/1 -> dout_0c_0/2(data_in)
  simple_bram_vacc_0_4/1(dout) -> pipeline92/1(d)
  simple_bram_vacc_0_4/2(valid) -> pipeline22/1(d)
  pipeline91/1(q) -> simple_bram_vacc_0_4/2(din)
  pipeline77/1(q) -> pipeline75/1(d)
  pipeline92/1(q) -> Reinterpret4/1
  pipeline22/1(q) -> dout_0c_0/3(we), Counter2/2
  pipeline75/1(q) -> Counter2/1
  pipeline76/1(q) -> simple_bram_vacc_0_4/1(new_acc)
  dout_0c_1/1(data_out) -> Terminator10/1
  Reinterpret1/1 -> dout_0c_1/2(data_in)
  simple_bram_vacc_0_5/1(dout) -> pipeline79/1(d)
  simple_bram_vacc_0_5/2(valid) -> pipeline80/1(d)
  pipeline78/1(q) -> simple_bram_vacc_0_5/2(din)
  pipeline79/1(q) -> Reinterpret1/1
  pipeline80/1(q) -> dout_0c_1/3(we)
  pipeline81/1(q) -> simple_bram_vacc_0_5/1(new_acc)
  dout_0c_2/1(data_out) -> Terminator11/1
  Reinterpret2/1 -> dout_0c_2/2(data_in)
  simple_bram_vacc_0_6/1(dout) -> pipeline83/1(d)
  simple_bram_vacc_0_6/2(valid) -> pipeline84/1(d)
  pipeline82/1(q) -> simple_bram_vacc_0_6/2(din)
  pipeline83/1(q) -> Reinterpret2/1
  pipeline84/1(q) -> dout_0c_2/3(we)
  pipeline85/1(q) -> simple_bram_vacc_0_6/1(new_acc)
  dout_0c_3/1(data_out) -> Terminator12/1
  Reinterpret3/1 -> dout_0c_3/2(data_in)
  simple_bram_vacc_0_7/1(dout) -> pipeline87/1(d)
  simple_bram_vacc_0_7/2(valid) -> pipeline88/1(d)
  pipeline86/1(q) -> simple_bram_vacc_0_7/2(din)
  pipeline87/1(q) -> Reinterpret3/1
  pipeline88/1(q) -> dout_0c_3/3(we)
  pipeline89/1(q) -> simple_bram_vacc_0_7/1(new_acc)
  Delay7/1 -> pipeline91/1(d)
  Delay8/1 -> pipeline78/1(d)
  Delay9/1 -> pipeline82/1(d)
  Delay10/1 -> pipeline86/1(d)
  Counter2/1 -> dout_0c_0/1(addr), dout_0c_1/1(addr), dout_0c_2/1(addr), dout_0c_3/1(addr)
  acc_cntrl1/1(new_acc) -> pipeline77/1(d), pipeline76/1(d), pipeline85/1(d), pipeline89/1(d), pipeline81/1(d)
  pipeline20/1 -> pipeline90/1(d), [fft1_sync]
  pipeline5/1 -> power4/1, [fft1_0]
  pipeline7/1 -> power5/1, [fft1_1]
  pipeline21/1 -> [fft1_2], power6/1
  pipeline93/1 -> [fft1_3], power7/1
  asiaa_adc5g0/12(c3) -> conv_bus1/4(i3)
  asiaa_adc5g0/14(c5) -> conv_bus1/6(i5)
  asiaa_adc5g0/10(c1) -> conv_bus1/2(i1)
  asiaa_adc5g0/13(c4) -> conv_bus1/5(i4)
  asiaa_adc5g0/9(c0) -> conv_bus1/1(i0)
  asiaa_adc5g0/15(c6) -> conv_bus1/7(i6)
  asiaa_adc5g0/16(c7) -> conv_bus1/8(i7)
  asiaa_adc5g0/11(c2) -> conv_bus1/3(i2)
  conv_bus0/6(q5) -> bus_create/6(in6), pipeline261/1
  pfb_fir_real0/9(pol1_out8) -> Delay24/1
  pfb_fir_real0/3(pol1_out2) -> Delay5/1
  pfb_fir_real0/4(pol1_out3) -> Delay16/1
  pfb_fir_real0/5(pol1_out4) -> Delay17/1
  pfb_fir_real0/6(pol1_out5) -> Delay21/1
  pfb_fir_real0/7(pol1_out6) -> Delay22/1
  pfb_fir_real0/8(pol1_out7) -> Delay23/1
  pfb_fir_real0/2(pol1_out1) -> Delay4/1
  pfb_fir_real1/5(pol1_out4) -> Delay28/1
  pfb_fir_real1/6(pol1_out5) -> Delay29/1
  pfb_fir_real1/7(pol1_out6) -> Delay30/1
  pfb_fir_real1/8(pol1_out7) -> Delay31/1
  pfb_fir_real1/9(pol1_out8) -> Delay33/1
  pfb_fir_real1/2(pol1_out1) -> Delay25/1
  pfb_fir_real1/3(pol1_out2) -> Delay26/1
  pfb_fir_real1/4(pol1_out3) -> Delay27/1
  Constant1/1 -> fft_wideband_real0/2(shift)
  Delay4/1 -> fft_wideband_real0/3(in00)
  Delay5/1 -> fft_wideband_real0/4(in01)
  Delay16/1 -> fft_wideband_real0/5(in02)
  Delay17/1 -> fft_wideband_real0/6(in03)
  Delay22/1 -> fft_wideband_real0/8(in05)
  Delay21/1 -> fft_wideband_real0/7(in04)
  Delay24/1 -> fft_wideband_real0/10(in07)
  Delay23/1 -> fft_wideband_real0/9(in06)
  Delay25/1 -> fft_wideband_real1/3(in00)
  Delay26/1 -> fft_wideband_real1/4(in01)
  Delay28/1 -> fft_wideband_real1/6(in03)
  Delay27/1 -> fft_wideband_real1/5(in02)
  Delay29/1 -> fft_wideband_real1/7(in04)
  Delay30/1 -> fft_wideband_real1/8(in05)
  Delay31/1 -> fft_wideband_real1/9(in06)
  Delay33/1 -> fft_wideband_real1/10(in07)
  power16/1 -> power_convert0/1(In1)
  power1/1 -> power_convert0/2(In2)
  power2/1 -> power_convert0/3(In3)
  power3/1 -> power_convert0/4(In4)
  power_convert0/1(Out1) -> Delay32/1
  power_convert0/2(Out2) -> Delay34/1
  power_convert0/3(Out3) -> Delay35/1
  power_convert0/4(Out4) -> Delay36/1
  pipeline72/1(q) -> pipeline127/1(d)
  pipeline127/1(q) -> acc_cntrl0/2(sync)
  power4/1 -> power_convert1/1(In1)
  power5/1 -> power_convert1/2(In2)
  power6/1 -> power_convert1/3(In3)
  power7/1 -> power_convert1/4(In4)
  power_convert1/1(Out1) -> Delay7/1
  power_convert1/2(Out2) -> Delay8/1
  power_convert1/3(Out3) -> Delay9/1
  power_convert1/4(Out4) -> Delay10/1
  pipeline90/1(q) -> pipeline128/1(d)
  pipeline128/1(q) -> acc_cntrl1/2(sync)
  Subsystem/2(din1_re) -> pipeline/1(d)
  Subsystem/3(din1_im) -> pipeline8/1(d)
  Subsystem/4(din2_re) -> pipeline13/1(d)
  Subsystem/5(din2_im) -> pipeline23/1(d)
  [cnt_rst] -> Register/2, acc_cntrl2/3(rst)
  [acc_doa] -> acc_cntrl2/1(acc_len)
  Subsystem/1(dout_sync) -> acc_cntrl2/2(sync), Logical/2
  pipeline/1(q) -> band_doa_correlator/1(din1_re)
  pipeline8/1(q) -> band_doa_correlator/2(din1_im)
  pipeline13/1(q) -> band_doa_correlator/3(din2_re)
  pipeline23/1(q) -> band_doa_correlator/4(din2_im)
  acc_cntrl2/1(new_acc) -> band_doa_correlator/6(new_acc)
  Logical/1 -> Register/1, Register/3
  Register/1 -> band_doa_correlator/5(din_valid)
  en_doa/1(in_reg) -> pipeline24/1(d)
  pipeline24/1(q) -> Slice2/1
  Slice2/1 -> [en_doa]
  [en_doa] -> Logical/1
  [fft0_sync] -> Subsystem/1(din_sync)
  [fft0_0] -> Subsystem/2(din1_0)
  [fft0_1] -> Subsystem/3(din1_1)
  [fft0_2] -> Subsystem/4(din1_2)
  [fft0_3] -> Subsystem/5(din1_3)
  [fft1_0] -> Subsystem/6(din2_0)
  [fft1_1] -> Subsystem/7(din2_1)
  [fft1_2] -> Subsystem/8(din2_2)
  [fft1_3] -> Subsystem/9(din2_3)
  Counter/1 -> r12/1(addr), r22/1(addr), r11/1(addr)
  band_doa_correlator/4(dout_valid) -> pipeline28/1
  band_doa_correlator/1(r11) -> pipeline25/1
  band_doa_correlator/2(r22) -> pipeline26/1
  band_doa_correlator/3(r12) -> pipeline27/1
  pipeline25/1 -> r11/2(data_in), conv_data/1(r11_in)
  pipeline26/1 -> conv_data/2(r22_in), r22/2(data_in)
  pipeline27/1 -> conv_data/3(r12_in), r12/2(data_in)
  pipeline28/1 -> r22/3(we), Counter/1, r11/3(we), r12/3(we), conv_data/4(din_valid)
  band_doa_correlator/5(band_num) -> pipeline29/1
  pipeline29/1 -> conv_data/5(band_in)
  conv_data/3(r12_out) -> doa_linalg/3(r12)
  conv_data/5(band_out) -> doa_linalg/5(band_in)
  conv_data/4(dout_valid) -> doa_linalg/4(din_valid)
  conv_data/1(r11_out) -> doa_linalg/1(r11)
  conv_data/2(r22_out) -> doa_linalg/2(r22)
  doa_linalg/9(fifo_full) -> gpio/1
  acc_doa/1(in_reg) -> pipeline30/1(d)
  pipeline30/1(q) -> [acc_doa]
  doa_linalg/1(lamb1) -> [l1]
  doa_linalg/2(lamb2) -> [l2]
  doa_linalg/3(eigen1_y) -> [eigen1]
  doa_linalg/4(eigen2_y) -> [eigen2]
  doa_linalg/5(eigen_x) -> [eigenx]
  doa_linalg/8(band_out) -> [band]
  Concat/1 -> Dual Port RAM/2
  [eigen1] -> Concat/1
  [eigen2] -> Concat/2
  [eigenx] -> Concat/3
  [band] -> Dual Port RAM/1
  doa_linalg/7(dout_error) -> Inverter/1
  Inverter/1 -> Logical1/2
  doa_linalg/6(dout_valid) -> Logical1/1
  Logical1/1 -> [doa_valid]
  [doa_valid] -> Dual Port RAM/3
  Constant/1 -> Dual Port RAM/6
  Constant2/1 -> Dual Port RAM/5
  doa_band/1(in_reg) -> Slice7/1
  [band_read] -> Dual Port RAM/4
  Dual Port RAM/1 -> Terminator2/1
  Dual Port RAM/2 -> Slice/1, Slice3/1, Slice4/1
  Slice/1 -> Convert2/1
  Slice3/1 -> Convert1/1
  Slice4/1 -> Convert/1
  Concat1/1 -> Dual Port RAM1/2
  [l1] -> Concat1/1
  [l2] -> Concat1/2
  [band] -> Dual Port RAM1/1
  [doa_valid] -> Dual Port RAM1/3
  Constant3/1 -> Dual Port RAM1/6
  Constant5/1 -> Dual Port RAM1/5
  [band_read] -> Dual Port RAM1/4
  Dual Port RAM1/1 -> Terminator3/1
  Dual Port RAM1/2 -> Slice5/1, Slice6/1
  Slice5/1 -> Convert4/1
  Slice6/1 -> Convert3/1
  Slice7/1 -> [band_read]
  Convert/1 -> eigen1/1(out_reg)
  Convert1/1 -> eigen2/1(out_reg)
  Convert2/1 -> eigenx/1(out_reg)
  Convert3/1 -> l1/1(out_reg)
  Convert4/1 -> l2/1(out_reg)
